simulink library "lib_all"  (Simulink 7.6)
settings: Solver=ode45  SolverMode=SingleTasking  StartTime=0.0  StopTime=10.0  RelTol=1e-3
values from neighbouring files (symbols this model uses):
  map_lib_03 = [6.8679 7.0159 7.4571 8.1849 9.1892 10.456 11.97 13.71 15.655 17.781 20.063 22.474 ... (803 elements, 73x11)]    [scr_simdata.mat]    (also in scr_simdata_all.mat)
  map_lib_06 = [2.5784 2.6727 2.9588 3.4453 4.1444 5.068 6.226 7.6242 9.2634 11.139 13.24 15.552 ... (803 elements, 73x11)]    [scr_simdata.mat]    (also in scr_simdata_all.mat)
  map_lib_09 = [0.0025784 0.0026727 0.0029588 0.0034453 0.0041444 0.005068 0.006226 0.0076242 0.0092634 0.011139 0.01324 0.015552 ... (803 elements, 73x11)]    [scr_simdata.mat]    (also in scr_simdata_all.mat)
  map_lib_10 = [2.74 2.75 2.76 2.77 2.78 2.79 2.8 2.81 2.82 2.83 2.84 2.85 ... (81 elements, 1x81)]    [scr_simdata.mat]    (also in scr_simdata_all.mat)
  map_lib_11 = [1.1983 1.2027 1.207 1.2113 1.2156 1.2198 1.2241 1.2283 1.2326 1.2368 1.241 1.2451 ... (81 elements, 1x81)]    [scr_simdata.mat]    (also in scr_simdata_all.mat)
  map_lib_12 = [2.74 2.75 2.76 2.77 2.78 2.79 2.8 2.81 2.82 2.83 2.84 2.85 ... (81 elements, 1x81)]    [scr_simdata.mat]    (also in scr_simdata_all.mat)
  map_lib_13 = [1.74 1.75 1.76 1.77 1.78 1.79 1.8 1.81 1.82 1.83 1.84 1.85 ... (81 elements, 1x81)]    [scr_simdata.mat]    (also in scr_simdata_all.mat)
  map_lib_16 = [0.00048828125 1.6159 1.8962 2.0821 2.2251 2.3426 2.4433 2.5318 2.611 2.683 2.749 2.8101 ... (1419 elements, 129x11)]    [scr_simdata.mat]    (also in scr_simdata_all.mat)
  map_mr2_01 = [0 800 1600 2400 3200 4000]    [scr_simdata.mat]    (also in scr_simdata_all.mat)
  map_mr2_02 = [1.32 1.32 1.33 1.34 1.36 1.38]    [scr_simdata.mat]    (also in scr_simdata_all.mat)
  map_mr2_03 = [0 800 1600 2400 3200 4000]    [scr_simdata.mat]    (also in scr_simdata_all.mat)
  map_mr2_04 = [1.32 1.32 1.33 1.34 1.36 1.38]    [scr_simdata.mat]    (also in scr_simdata_all.mat)
  map_mr2_05 = [0 800 1600 2400 3200 4000]    [scr_simdata.mat]    (also in scr_simdata_all.mat)
  map_mr2_06 = [1.32 1.32 1.33 1.34 1.36 1.38]    [scr_simdata.mat]    (also in scr_simdata_all.mat)
  Parameter1 = 6    [scr_simdata.mat]    (also in scr_simdata_all.mat)
  Parameter101 = 30    [scr_simdata.mat]    (also in scr_simdata_all.mat)
  Parameter102 = 12    [scr_simdata.mat]    (also in scr_simdata_all.mat)
  Parameter103 = -240    [scr_simdata.mat]    (also in scr_simdata_all.mat)
  Parameter108 = 48    [scr_simdata.mat]    (also in scr_simdata_all.mat)
  Parameter120 = 16    [scr_simdata.mat]    (also in scr_simdata_all.mat)
  Parameter121 = 87    [scr_simdata.mat]    (also in scr_simdata_all.mat)
  Parameter123 = 78    [scr_simdata.mat]    (also in scr_simdata_all.mat)
  Parameter124 = 60    [scr_simdata.mat]    (also in scr_simdata_all.mat)
  Parameter125 = -480    [scr_simdata.mat]    (also in scr_simdata_all.mat)
  Parameter130 = 96    [scr_simdata.mat]    (also in scr_simdata_all.mat)
  Parameter131 = 3    [scr_simdata.mat]    (also in scr_simdata_all.mat)
  Parameter144 = 3    [scr_simdata.mat]    (also in scr_simdata_all.mat)
  Parameter18 = 128    [scr_simdata.mat]    (also in scr_simdata_all.mat)
  Parameter19 = 16    [scr_simdata.mat]    (also in scr_simdata_all.mat)
  Parameter2 = 12    [scr_simdata.mat]    (also in scr_simdata_all.mat)
  Parameter20 = 7    [scr_simdata.mat]    (also in scr_simdata_all.mat)
  Parameter21 = 4    [scr_simdata.mat]    (also in scr_simdata_all.mat)
  Parameter3 = 18    [scr_simdata.mat]    (also in scr_simdata_all.mat)
  Parameter32 = 1    [scr_simdata.mat]    (also in scr_simdata_all.mat)
  Parameter34 = 24    [scr_simdata.mat]    (also in scr_simdata_all.mat)
  Parameter4 = 138    [scr_simdata.mat]    (also in scr_simdata_all.mat)
  Parameter42 = 48    [scr_simdata.mat]    (also in scr_simdata_all.mat)
  Parameter5 = 135    [scr_simdata.mat]    (also in scr_simdata_all.mat)
  Parameter55 = 0    [scr_simdata.mat]    (also in scr_simdata_all.mat)
  Parameter56 = 0    [scr_simdata.mat]    (also in scr_simdata_all.mat)
  Parameter57 = 1    [scr_simdata.mat]    (also in scr_simdata_all.mat)
  Parameter58 = 1    [scr_simdata.mat]    (also in scr_simdata_all.mat)
  Parameter59 = 2    [scr_simdata.mat]    (also in scr_simdata_all.mat)
  Parameter6 = 132    [scr_simdata.mat]    (also in scr_simdata_all.mat)
  Parameter60 = 2    [scr_simdata.mat]    (also in scr_simdata_all.mat)
  Parameter63 = 144    [scr_simdata.mat]    (also in scr_simdata_all.mat)
  Parameter7 = 126    [scr_simdata.mat]    (also in scr_simdata_all.mat)
  Parameter76 = 64    [scr_simdata.mat]    (also in scr_simdata_all.mat)
  Parameter77 = 135    [scr_simdata.mat]    (also in scr_simdata_all.mat)
  Parameter79 = 126    [scr_simdata.mat]    (also in scr_simdata_all.mat)
  Parameter80 = 108    [scr_simdata.mat]    (also in scr_simdata_all.mat)
  Parameter81 = 0    [scr_simdata.mat]    (also in scr_simdata_all.mat)
  Parameter86 = 0    [scr_simdata.mat]    (also in scr_simdata_all.mat)
  Parameter98 = 112    [scr_simdata.mat]    (also in scr_simdata_all.mat)
  Parameter99 = 39    [scr_simdata.mat]    (also in scr_simdata_all.mat)
undefined symbols (not defined in the file or in its folder): cylvolkp_hih_map2h, cylvolkp_map2h, pk1k_map2h
libraries: simulink x2
(part 15 of 36)

== system lib_all/SubSystem7/SubSystem134/SubSystem136/SubSystem139 ==
  Block323 : Inport
  Block324 : Inport  Port=2
  Block325 : Inport  Port=3
  Block326 : Constant  Value=Parameter20  OutDataTypeStr=double  SampleTime=-1
  Block327 : Constant  Value=2  OutDataTypeStr=double  SampleTime=-1
  Block328 : Constant  Value=0  OutDataTypeStr=double  SampleTime=-1
  Block329 : Math  Operator=pow  SaturateOnIntegerOverflow=on
  Block330 : Product  Inputs=*/  OutDataTypeStr=double  SaturateOnIntegerOverflow=on  RndMeth=Zero  InputSameDT=on
  Block331 : Product  Inputs=*/  OutDataTypeStr=double  SaturateOnIntegerOverflow=on  RndMeth=Zero  InputSameDT=on
  Block332 : Sum  Inputs=-+  OutDataTypeStr=double  RndMeth=Zero  SaturateOnIntegerOverflow=on  InputSameDT=on
  SubSystem140 : Subsystem
  SubSystem141 : Subsystem  Priority=1  TreatAsAtomicUnit=on  mask(***ATC.DSWVEC***){bd_version=1.01.A, r0=DSM371, r1=-1, r2=, r3=, r4=, r5=, r6=, r7=, r8=, r9=, c0=, c1=, c2=, c3=, c4=, c5=, c6=, c7=, c8=, c9=}
      mask prompts: bd_version "Version"
      mask help: これはAutoCからのブロックです．編集禁止．
      settings: AttributesFormatString=Attribute62  OpenFcn=warndlg('変更禁止!');
      mask Help: web(ui_blockhelpmng(gcb));
      mask Display: disp('ATC.DSRVEC')
  SubSystem142 : Subsystem  Priority=2  TreatAsAtomicUnit=on  mask(***ATC.DSWVEC***){bd_version=1.01.A, r0=DSM373, r1=-1, r2=, r3=, r4=, r5=, r6=, r7=, r8=, r9=, c0=, c1=, c2=, c3=, c4=, c5=, c6=, c7=, c8=, c9=}
      mask prompts: bd_version "Version"
      mask help: これはAutoCからのブロックです．編集禁止．
      settings: AttributesFormatString=Attribute63  OpenFcn=warndlg('変更禁止!');
      mask Help: web(ui_blockhelpmng(gcb));
      mask Display: disp('ATC.DSRVEC')
  SubSystem143 : Subsystem  Priority=3  TreatAsAtomicUnit=on  mask(***ATC.DSWVEC***){bd_version=1.01.A, r0=DSM375, r1=-1, r2=, r3=, r4=, r5=, r6=, r7=, r8=, r9=, c0=, c1=, c2=, c3=, c4=, c5=, c6=, c7=, c8=, c9=}
      mask prompts: bd_version "Version"
      mask help: これはAutoCからのブロックです．編集禁止．
      settings: AttributesFormatString=Attribute64  OpenFcn=warndlg('変更禁止!');
      mask Help: web(ui_blockhelpmng(gcb));
      mask Display: disp('ATC.DSRVEC')
  SubSystem144 : Subsystem  Priority=4
      settings: AttributesFormatString=Attribute65
  wires:
  Block327/1 -> Block329/1
  Block326/1 -> Block329/2
  Block330/1 -> SubSystem141/1(input), Block332/2
  Block331/1 -> SubSystem142/1(input), Block332/1
  Block323/1 -> Block330/1
  Block325/1 -> SubSystem141/2(element), SubSystem143/2(element), SubSystem142/2(element)
  Block324/1 -> Block331/1
  Block332/1 -> SubSystem143/1(input), SubSystem140/1(In1)
  Block328/1 -> SubSystem140/2(In2)
  SubSystem140/1(Out1) -> SubSystem144/Enable
  Block329/1 -> Block330/2, Block331/2

== system lib_all/SubSystem7/SubSystem134/SubSystem136/SubSystem149 ==
  Block343 : Inport
  Block344 : Inport  Port=2
  Block345 : TriggerPort  TriggerType=function-call
  Block346 : Constant  OutDataTypeStr=double  SampleTime=-1
  Block347 : Sum  OutDataTypeStr=double  RndMeth=Zero  SaturateOnIntegerOverflow=on  InputSameDT=on
  Block348 : Sum  OutDataTypeStr=double  RndMeth=Zero  SaturateOnIntegerOverflow=on  InputSameDT=on
  Block349 : Switch  Threshold=0.5  RndMeth=Zero  SaturateOnIntegerOverflow=on  InputSameDT=on
  Block350 : Switch  Threshold=0.5  RndMeth=Zero  SaturateOnIntegerOverflow=on  InputSameDT=on
  Block351 : UnitDelay  SampleTime=-1
  Block352 : UnitDelay  SampleTime=-1
  SubSystem150 : Subsystem
  SubSystem151 : Subsystem
  SubSystem155 : Subsystem
  Block361 : Outport
  Block362 : Outport  Port=2
  wires:
  Block343/1 -> SubSystem151/2(Block354), SubSystem155/2(Block358), SubSystem150/1(In1)
  Block346/1 -> SubSystem150/2(In2)
  SubSystem150/1(Out1) -> Block349/2, Block350/2
  Block349/1 -> Block361/1, Block351/1
  Block344/1 -> SubSystem151/1(Block353), SubSystem155/1(Block357)
  Block347/1 -> Block349/3
  SubSystem151/1(Block356) -> Block347/1, Block349/1
  Block351/1 -> Block347/2
  Block350/1 -> Block362/1, Block352/1
  Block348/1 -> Block350/3
  SubSystem155/1(Block360) -> Block348/1, Block350/1
  Block352/1 -> Block348/2

== system lib_all/SubSystem7/SubSystem161/SubSystem164/SubSystem165 ==
  note: ***編集禁止***
  N : Inport
  For_loop : Stateflow chart  TreatAsAtomicUnit=on
  action : Outport
  i : Outport  Port=2
  wires:
  For_loop/2(function_call) -> action/1
  N/1 -> For_loop/1(N_Loop)
  For_loop/1(idx) -> i/1

== system lib_all/SubSystem7/SubSystem161/SubSystem164/SubSystem167 ==
  note: ***編集禁止***
  N : Inport
  For_loop : Stateflow chart  TreatAsAtomicUnit=on
  action : Outport
  i : Outport  Port=2
  wires:
  For_loop/2(function_call) -> action/1
  N/1 -> For_loop/1(N_Loop)
  For_loop/1(idx) -> i/1

== system lib_all/SubSystem7/SubSystem161/SubSystem164/SubSystem169 ==
  Block375 : Inport
  Block376 : TriggerPort  TriggerType=function-call
  Block377 : Constant  OutDataTypeStr=double  SampleTime=-1
  Block378 : Product  Inputs=*/  OutDataTypeStr=double  SaturateOnIntegerOverflow=on  RndMeth=Zero  InputSameDT=on
  SubSystem170 : Subsystem  TreatAsAtomicUnit=on  mask(***ATC.DSWVEC***){bd_version=1.01.A, r0=DSM87, r1=-1, r2=, r3=, r4=, r5=, r6=, r7=, r8=, r9=, c0=, c1=, c2=, c3=, c4=, c5=, c6=, c7=, c8=, c9=}
      mask prompts: bd_version "Version"
      mask help: これはAutoCからのブロックです．編集禁止．
      settings: OpenFcn=warndlg('変更禁止!');
      mask Help: web(ui_blockhelpmng(gcb));
      mask Display: disp('ATC.DSRVEC')
  SubSystem171 : Subsystem  TreatAsAtomicUnit=on  mask(***ATC.DSRVEC***){bd_version=1.01.A, r0=DSM375, r1=-1, r2=, r3=, r4=, r5=, r6=, r7=, r8=, r9=, c0=, c1=, c2=, c3=, c4=, c5=, c6=, c7=, c8=, c9=}
      mask prompts: bd_version "Version"
      mask help: これはAutoCからのブロックです．編集禁止．
      settings: OpenFcn=warndlg('変更禁止!');
      mask Help: web(ui_blockhelpmng(gcb));
      mask Display: disp('ATC.DSRVEC')
  SubSystem172 : Subsystem  TreatAsAtomicUnit=on  mask(***ATC.DSRVEC***){bd_version=1.01.A, r0=DSM375, r1=-1, r2=, r3=, r4=, r5=, r6=, r7=, r8=, r9=, c0=, c1=, c2=, c3=, c4=, c5=, c6=, c7=, c8=, c9=}
      mask prompts: bd_version "Version"
      mask help: これはAutoCからのブロックです．編集禁止．
      settings: OpenFcn=warndlg('変更禁止!');
      mask Help: web(ui_blockhelpmng(gcb));
      mask Display: disp('ATC.DSRVEC')
  wires:
  Block377/1 -> SubSystem172/1(element)
  Block375/1 -> SubSystem170/2(element), SubSystem171/1(element)
  SubSystem172/1(Output) -> Block378/1
  SubSystem171/1(Output) -> Block378/2
  Block378/1 -> SubSystem170/1(input)

== system lib_all/SubSystem7/SubSystem161/SubSystem164/SubSystem173 ==
  Block379 : Inport
  Block380 : TriggerPort  TriggerType=function-call
  Block381 : Constant  Value=0  OutDataTypeStr=double  SampleTime=-1
  SubSystem174 : Subsystem  TreatAsAtomicUnit=on  mask(***ATC.DSWVEC***){bd_version=1.01.A, r0=DSM374, r1=-1, r2=, r3=, r4=, r5=, r6=, r7=, r8=, r9=, c0=, c1=, c2=, c3=, c4=, c5=, c6=, c7=, c8=, c9=}
      mask prompts: bd_version "Version"
      mask help: これはAutoCからのブロックです．編集禁止．
      settings: OpenFcn=warndlg('変更禁止!');
      mask Help: web(ui_blockhelpmng(gcb));
      mask Display: disp('ATC.DSRVEC')
  wires:
  Block379/1 -> SubSystem174/2(element)
  Block381/1 -> SubSystem174/1(input)

== system lib_all/SubSystem7/SubSystem161/SubSystem164/SubSystem175 ==
  Block382 : Constant  Value=0  OutDataTypeStr=double  SampleTime=-1
  Block383 : DataStoreWrite  DataStoreName=DSM365
  wires:
  Block382/1 -> Block383/1

== system lib_all/SubSystem7/SubSystem9/SubSystem13/SubSystem120 ==
  note: Text16
  Block283 : Inport
  Block284 : Inport  Port=2
  Block285 : Inport  Port=3
  Block286 : SwitchCase  CaseConditions={1 2 3}
  Block287 : Terminator
  SubSystem121 : Subsystem
      settings: RTWSystemCode=Inline
  SubSystem123 : Subsystem
      settings: RTWSystemCode=Inline
  SubSystem125 : Subsystem
      settings: RTWSystemCode=Inline
  wires:
  Block284/1 -> Block286/1
  Block285/1 -> SubSystem121/2(Block289), SubSystem123/2(Block292), SubSystem125/2(Block295)
  Block283/1 -> SubSystem121/1(Block288), SubSystem123/1(Block291), SubSystem125/1(Block294)
  Block286/1 -> SubSystem121/Action
  Block286/2 -> SubSystem123/Action
  Block286/3 -> SubSystem125/Action
  Block286/4 -> Block287/1

== system lib_all/SubSystem7/SubSystem9/SubSystem13/SubSystem127 ==
  note: Text17
  Block297 : Inport
  Block298 : Inport  Port=2
  Block299 : Inport  Port=3
  Block300 : SwitchCase  CaseConditions={1 2 3}
  Block301 : Terminator
  SubSystem128 : Subsystem
      settings: RTWSystemCode=Inline
  SubSystem130 : Subsystem
      settings: RTWSystemCode=Inline
  SubSystem132 : Subsystem
      settings: RTWSystemCode=Inline
  wires:
  Block300/2 -> SubSystem130/Action
  Block300/1 -> SubSystem128/Action
  Block299/1 -> SubSystem130/2(Block306), SubSystem132/2(Block309), SubSystem128/2(Block303)
  Block297/1 -> SubSystem130/1(Block305), SubSystem132/1(Block308), SubSystem128/1(Block302)
  Block298/1 -> Block300/1
  Block300/3 -> SubSystem132/Action
  Block300/4 -> Block301/1

== system lib_all/SubSystem7/SubSystem9/SubSystem13/SubSystem14 ==
  note: Text9
  Block39 : Inport
  Block40 : Inport  Port=2
  Block41 : Inport  Port=3
  Block42 : Inport  Port=4
  Block43 : Constant  Value=0  OutDataTypeStr=double  SampleTime=-1
  Block44 : Constant  OutDataTypeStr=double  SampleTime=-1
  Block45 : Constant  Value=0  OutDataTypeStr=double  SampleTime=-1
  Block46 : Gain  Gain=1/5  SaturateOnIntegerOverflow=on  OutDataTypeStr=Inherit: Same as input  ParamDataTypeStr=Inherit: Same as input
      settings: AttributesFormatString=Attribute20
  Block47 : Product  OutDataTypeStr=double  SaturateOnIntegerOverflow=on  RndMeth=Zero  InputSameDT=on
  Block48 : Sum  Inputs=-+  OutDataTypeStr=double  RndMeth=Zero  SaturateOnIntegerOverflow=on  InputSameDT=on
  Block49 : Sum  OutDataTypeStr=double  RndMeth=Zero  SaturateOnIntegerOverflow=on  InputSameDT=on
  Block50 : Sum  OutDataTypeStr=double  RndMeth=Zero  SaturateOnIntegerOverflow=on  InputSameDT=on
  Block51 : Switch  Threshold=0.5  RndMeth=Zero  SaturateOnIntegerOverflow=on  InputSameDT=on
  SubSystem16 : Subsystem
  SubSystem17 : Subsystem
  SubSystem23 : Subsystem
  Block160 : Outport
  wires:
  Block50/1 -> SubSystem23/3(Block81), SubSystem17/2(Block53)
  Block39/1 -> Block50/1
  Block46/1 -> Block49/2
  Block47/1 -> Block46/1
  Block44/1 -> Block50/2
  Block43/1 -> Block51/1
  Block51/1 -> Block160/1
  SubSystem16/1(Out1) -> Block51/2
  Block45/1 -> SubSystem16/2(In2)
  Block41/1 -> SubSystem17/3(Block54)
  Block49/1 -> SubSystem16/1(In1), Block51/3
  SubSystem17/2(Block78) -> Block47/2
  Block48/1 -> Block47/1  "SigLabel3"
  SubSystem23/2(Block159) -> Block48/2
  SubSystem23/1(Block158) -> Block48/1, Block49/1
  Block42/1 -> SubSystem23/2(Block80)
  SubSystem17/1(Block77) -> SubSystem23/1(Block79)
  Block40/1 -> SubSystem17/1(Block52)

== system lib_all/SubSystem7/SubSystem9/SubSystem13/SubSystem67 ==
  note: Text15
  Block161 : Inport
  Block162 : Inport  Port=2
  Block163 : Inport  Port=3
  Block164 : Inport  Port=4
  Block165 : Constant  Value=0  OutDataTypeStr=double  SampleTime=-1
  Block166 : Constant  OutDataTypeStr=double  SampleTime=-1
  Block167 : Constant  Value=0  OutDataTypeStr=double  SampleTime=-1
  Block168 : Gain  Gain=1/5  SaturateOnIntegerOverflow=on  OutDataTypeStr=Inherit: Same as input  ParamDataTypeStr=Inherit: Same as input
      settings: AttributesFormatString=Attribute39
  Block169 : Product  OutDataTypeStr=double  SaturateOnIntegerOverflow=on  RndMeth=Zero  InputSameDT=on
  Block170 : Sum  Inputs=-+  OutDataTypeStr=double  RndMeth=Zero  SaturateOnIntegerOverflow=on  InputSameDT=on
  Block171 : Sum  OutDataTypeStr=double  RndMeth=Zero  SaturateOnIntegerOverflow=on  InputSameDT=on
  Block172 : Sum  OutDataTypeStr=double  RndMeth=Zero  SaturateOnIntegerOverflow=on  InputSameDT=on
  Block173 : Switch  Threshold=0.5  RndMeth=Zero  SaturateOnIntegerOverflow=on  InputSameDT=on
  SubSystem69 : Subsystem
  SubSystem70 : Subsystem
  SubSystem76 : Subsystem
  Block282 : Outport
  wires:
  Block172/1 -> SubSystem76/3(Block203), SubSystem70/2(Block175)
  Block161/1 -> Block172/1
  Block168/1 -> Block171/2
  Block169/1 -> Block168/1
  Block166/1 -> Block172/2
  Block165/1 -> Block173/1
  Block173/1 -> Block282/1
  SubSystem69/1(Out1) -> Block173/2
  Block167/1 -> SubSystem69/2(In2)
  Block163/1 -> SubSystem70/3(Block176)
  Block171/1 -> SubSystem69/1(In1), Block173/3
  SubSystem70/2(Block200) -> Block169/2
  Block170/1 -> Block169/1  "SigLabel6"
  SubSystem76/2(Block281) -> Block170/2
  SubSystem76/1(Block280) -> Block170/1, Block171/1
  Block164/1 -> SubSystem76/2(Block202)
  SubSystem70/1(Block199) -> SubSystem76/1(Block201)
  Block162/1 -> SubSystem70/1(Block174)

== system lib_all/SubSystem727/SubSystem740/SubSystem743/SubSystem744 ==
  Block1737 : Inport
  Block1738 : Inport  Port=2
  Block1739 : Constant  Value=Parameter32  OutDataTypeStr=double  SampleTime=-1
  Block1740 : Constant  Value=2  OutDataTypeStr=double  SampleTime=-1
  Block1741 : Constant  Value=Parameter42  OutDataTypeStr=double  SampleTime=-1
  Block1742 : Constant  Value=0  OutDataTypeStr=double  SampleTime=-1
  Block1743 : Constant  Value=72  OutDataTypeStr=double  SampleTime=-1
  Block1744 : Constant  Value=Parameter34  OutDataTypeStr=double  SampleTime=-1
  Block1745 : Logic  Operator=OR
  Block1746 : Sum  Inputs=+-  OutDataTypeStr=double  RndMeth=Zero  SaturateOnIntegerOverflow=on  InputSameDT=on
  Block1747 : Switch  Threshold=0.5  RndMeth=Zero  SaturateOnIntegerOverflow=on  InputSameDT=on
  Block1748 : Switch  Threshold=0.5  RndMeth=Zero  SaturateOnIntegerOverflow=on  InputSameDT=on
  SubSystem745 : Subsystem
  SubSystem746 : Subsystem
  SubSystem747 : Subsystem
      settings: RTWSystemCode=Inline
  Block1755 : Outport
  wires:
  Block1748/1 -> Block1755/1
  Block1747/1 -> Block1746/2
  Block1744/1 -> Block1747/3
  Block1741/1 -> Block1747/1
  Block1745/1 -> Block1748/2
  SubSystem746/1(Out1) -> Block1745/2
  SubSystem745/1(Out1) -> Block1747/2, Block1745/1
  Block1740/1 -> SubSystem746/2(In2)
  Block1739/1 -> SubSystem745/1(In1)
  Block1738/1 -> SubSystem746/1(In1), SubSystem745/2(In2)
  Block1737/1 -> Block1746/1, Block1748/3
  Block1746/1 -> SubSystem747/2(Block1750)
  Block1742/1 -> SubSystem747/3(Block1751)
  Block1743/1 -> SubSystem747/1(Block1749)
  SubSystem747/1(Block1754) -> Block1748/1

== system lib_all/SubSystem727/SubSystem740/SubSystem743/SubSystem749 ==
  Block1756 : Inport
  Block1757 : Inport  Port=2
  Block1758 : Inport  Port=3
  Block1759 : Sum  OutDataTypeStr=double  RndMeth=Zero  SaturateOnIntegerOverflow=on  InputSameDT=on
  Block1760 : Switch  Threshold=0.5  RndMeth=Zero  SaturateOnIntegerOverflow=on  InputSameDT=on
  SubSystem750 : Subsystem
  Block1761 : Outport
  wires:
  Block1756/1 -> Block1759/1
  Block1760/1 -> Block1761/1
  Block1759/1 -> Block1760/1
  SubSystem750/1(Out1) -> Block1760/2
  Block1758/1 -> SubSystem750/2(In2)
  Block1757/1 -> Block1759/2, Block1760/3, SubSystem750/1(In1)

== system lib_all/SubSystem727/SubSystem740/SubSystem752/SubSystem753 ==
  note: ***編集禁止***
  In1 : Inport
  In2 : Inport  Port=2
  RO1 : RelationalOperator  Operator=>  InputSameDT=on
  single1 : DataTypeConversion  OutDataTypeStr=single  SaturateOnIntegerOverflow=on  RndMeth=Zero
  single2 : DataTypeConversion  OutDataTypeStr=single  SaturateOnIntegerOverflow=on  RndMeth=Zero
  Out1 : Outport
  wires:
  RO1/1 -> Out1/1
  In2/1 -> single2/1
  In1/1 -> single1/1
  single1/1 -> RO1/1
  single2/1 -> RO1/2

== system lib_all/SubSystem727/SubSystem754/SubSystem756/SubSystem757 ==
  note: ***編集禁止***
  mult : Inport
  div : Inport  Port=2
  init : Inport  Port=3
  Product : Subsystem  TreatAsAtomicUnit=on
      settings: RTWSystemCode=Inline
  Out : Outport
  wires:
  Product/1(Out) -> Out/1
  mult/1 -> Product/1(mult)
  div/1 -> Product/2(div)
  init/1 -> Product/3(init)

== system lib_all/SubSystem727/SubSystem754/SubSystem756/SubSystem758 ==
  note: ***編集禁止***
  element : Inport
  Inside_DSR : DataStoreRead  DataStoreName=DSM95
  Selector : Selector  IndexOptions=Index vector (port)  Indices=[1 3]  OutputSizes=1
  Output : Outport
  wires:
  Selector/1 -> Output/1
  element/1 -> Selector/2
  Inside_DSR/1 -> Selector/1

== system lib_all/SubSystem727/SubSystem754/SubSystem756/SubSystem759 ==
  Block1804 : Inport
  Block1805 : Inport  Port=2
  Block1806 : Inport  Port=3
  Block1807 : Sum  OutDataTypeStr=double  RndMeth=Zero  SaturateOnIntegerOverflow=on  InputSameDT=on
  Block1808 : Sum  Inputs=+-  OutDataTypeStr=double  RndMeth=Zero  SaturateOnIntegerOverflow=on  InputSameDT=on
  Block1809 : Switch  Threshold=0.5  RndMeth=Zero  SaturateOnIntegerOverflow=on  InputSameDT=on
  Block1810 : Switch  Threshold=0.5  RndMeth=Zero  SaturateOnIntegerOverflow=on  InputSameDT=on
  SubSystem760 : Subsystem
  SubSystem761 : Subsystem
  Block1811 : Outport
  wires:
  Block1805/1 -> SubSystem760/1(In1), Block1808/1, SubSystem761/1(In1), Block1810/3, Block1807/2
  Block1804/1 -> Block1807/1, Block1808/2, SubSystem760/2(In2)
  SubSystem760/1(Out1) -> Block1809/2
  Block1806/1 -> SubSystem761/2(In2)
  SubSystem761/1(Out1) -> Block1810/2
  Block1810/1 -> Block1809/3
  Block1807/1 -> Block1810/1
  Block1809/1 -> Block1811/1
  Block1808/1 -> Block1809/1

== system lib_all/SubSystem727/SubSystem754/SubSystem762/SubSystem763 ==
  note: ***編集禁止***
  In1 : Inport
  In2 : Inport  Port=2
  LO1 : Logic
  RO1 : RelationalOperator  Operator=<  InputSameDT=on
  RO2 : RelationalOperator  Operator=~=  InputSameDT=on
  single1 : DataTypeConversion  OutDataTypeStr=single  SaturateOnIntegerOverflow=on  RndMeth=Zero
  single2 : DataTypeConversion  OutDataTypeStr=single  SaturateOnIntegerOverflow=on  RndMeth=Zero
  Out1 : Outport
  wires:
  LO1/1 -> Out1/1
  RO2/1 -> LO1/2
  RO1/1 -> LO1/1
  In2/1 -> single2/1
  In1/1 -> single1/1
  single1/1 -> RO2/1, RO1/1
  single2/1 -> RO2/2, RO1/2

== system lib_all/SubSystem727/SubSystem754/SubSystem762/SubSystem764 ==
  note: ***編集禁止***
  In1 : Inport
  In2 : Inport  Port=2
  RO1 : RelationalOperator  Operator===  InputSameDT=on
  single1 : DataTypeConversion  OutDataTypeStr=single  SaturateOnIntegerOverflow=on  RndMeth=Zero
  single2 : DataTypeConversion  OutDataTypeStr=single  SaturateOnIntegerOverflow=on  RndMeth=Zero
  Out1 : Outport
  wires:
  RO1/1 -> Out1/1
  In2/1 -> single2/1
  In1/1 -> single1/1
  single1/1 -> RO1/1
  single2/1 -> RO1/2

== system lib_all/SubSystem727/SubSystem754/SubSystem762/SubSystem765 ==
  note: ***編集禁止***
  In1 : Inport
  In2 : Inport  Port=2
  RO1 : RelationalOperator  Operator===  InputSameDT=on
  single1 : DataTypeConversion  OutDataTypeStr=single  SaturateOnIntegerOverflow=on  RndMeth=Zero
  single2 : DataTypeConversion  OutDataTypeStr=single  SaturateOnIntegerOverflow=on  RndMeth=Zero
  Out1 : Outport
  wires:
  RO1/1 -> Out1/1
  In2/1 -> single2/1
  In1/1 -> single1/1
  single1/1 -> RO1/1
  single2/1 -> RO1/2

== system lib_all/SubSystem727/SubSystem754/SubSystem762/SubSystem766 ==
  Block1832 : Inport
  Block1833 : Inport  Port=2
  Block1834 : EnablePort
  Block1835 : Demux  Outputs=2
  Block1836 : Mux  Inputs=2
  SubSystem767 : Subsystem
  SubSystem771 : Subsystem
  Block1845 : Outport
  wires:
  Block1832/1 -> Block1835/1
  Block1833/1 -> SubSystem771/1(Block1841), SubSystem767/1(Block1837)
  Block1835/1 -> SubSystem767/2(Block1838)
  Block1835/2 -> SubSystem771/2(Block1842)
  SubSystem767/1(Block1840) -> Block1836/1
  SubSystem771/1(Block1844) -> Block1836/2
  Block1836/1 -> Block1845/1

== system lib_all/SubSystem727/SubSystem754/SubSystem762/SubSystem775 ==
  Block1846 : Inport
  Block1847 : Inport  Port=2
  Block1848 : EnablePort
  Block1849 : Demux  Outputs=2
  Block1850 : Mux  Inputs=2
  Block1851 : Sum  OutDataTypeStr=double  RndMeth=Zero  SaturateOnIntegerOverflow=on  InputSameDT=on
  SubSystem776 : Subsystem  TreatAsAtomicUnit=on  mask(***ATC.DSRVEC***){bd_version=1.01.A, r0=DSM4, r1=-1, r2=, r3=, r4=, r5=, r6=, r7=, r8=, r9=, c0=, c1=, c2=, c3=, c4=, c5=, c6=, c7=, c8=, c9=}
      mask prompts: bd_version "Version"
      mask help: これはAutoCからのブロックです．編集禁止．
      settings: OpenFcn=warndlg('変更禁止!');
      mask Help: web(ui_blockhelpmng(gcb));
      mask Display: disp('ATC.DSRVEC')
  SubSystem777 : Subsystem
  SubSystem781 : Subsystem
  Block1860 : Outport
  wires:
  Block1849/2 -> SubSystem781/2(Block1857)
  Block1849/1 -> SubSystem777/2(Block1853)
  Block1846/1 -> Block1849/1
  SubSystem777/1(Block1855) -> Block1850/1
  SubSystem781/1(Block1859) -> Block1850/2
  Block1851/1 -> Block1860/1
  SubSystem776/1(Output) -> Block1851/1
  Block1850/1 -> Block1851/2
  Block1847/1 -> SubSystem781/1(Block1856), SubSystem777/1(Block1852), SubSystem776/1(element)

== system lib_all/SubSystem727/SubSystem754/SubSystem762/SubSystem785 ==
  Block1861 : Inport
  Block1862 : Inport  Port=2
  Block1863 : EnablePort
  Block1864 : Demux  Outputs=2
  Block1865 : Mux  Inputs=2
  Block1866 : Sum  OutDataTypeStr=double  RndMeth=Zero  SaturateOnIntegerOverflow=on  InputSameDT=on
  SubSystem786 : Subsystem  TreatAsAtomicUnit=on  mask(***ATC.DSRVEC***){bd_version=1.01.A, r0=DSM2, r1=-1, r2=, r3=, r4=, r5=, r6=, r7=, r8=, r9=, c0=, c1=, c2=, c3=, c4=, c5=, c6=, c7=, c8=, c9=}
      mask prompts: bd_version "Version"
      mask help: これはAutoCからのブロックです．編集禁止．
      settings: OpenFcn=warndlg('変更禁止!');
      mask Help: web(ui_blockhelpmng(gcb));
      mask Display: disp('ATC.DSRVEC')
  SubSystem787 : Subsystem
  SubSystem791 : Subsystem
  Block1875 : Outport
  wires:
  Block1861/1 -> Block1864/1
  Block1864/1 -> SubSystem787/2(Block1868)
  Block1864/2 -> SubSystem791/2(Block1872)
  SubSystem787/1(Block1870) -> Block1865/1
  SubSystem791/1(Block1874) -> Block1865/2
  Block1866/1 -> Block1875/1
  Block1865/1 -> Block1866/2
  Block1862/1 -> SubSystem791/1(Block1871), SubSystem787/1(Block1867), SubSystem786/1(element)
  SubSystem786/1(Output) -> Block1866/1

== system lib_all/SubSystem800/SubSystem803/SubSystem804/SubSystem805 ==
  note: ***編集禁止***
  In1 : Inport
  In2 : Inport  Port=2
  RO1 : RelationalOperator  Operator=>  InputSameDT=on
  single1 : DataTypeConversion  OutDataTypeStr=single  SaturateOnIntegerOverflow=on  RndMeth=Zero
  single2 : DataTypeConversion  OutDataTypeStr=single  SaturateOnIntegerOverflow=on  RndMeth=Zero
  Out1 : Outport
  wires:
  single2/1 -> RO1/2
  single1/1 -> RO1/1
  In1/1 -> single1/1
  In2/1 -> single2/1
  RO1/1 -> Out1/1

== system lib_all/SubSystem800/SubSystem803/SubSystem804/SubSystem806 ==
  note: ***編集禁止***
  In1 : Inport
  In2 : Inport  Port=2
  RO1 : RelationalOperator  Operator=>  InputSameDT=on
  single1 : DataTypeConversion  OutDataTypeStr=single  SaturateOnIntegerOverflow=on  RndMeth=Zero
  single2 : DataTypeConversion  OutDataTypeStr=single  SaturateOnIntegerOverflow=on  RndMeth=Zero
  Out1 : Outport
  wires:
  RO1/1 -> Out1/1
  In2/1 -> single2/1
  In1/1 -> single1/1
  single1/1 -> RO1/1
  single2/1 -> RO1/2

== system lib_all/SubSystem800/SubSystem803/SubSystem804/SubSystem807 ==
  note: ***編集禁止***
  In1 : Inport
  In2 : Inport  Port=2
  LO1 : Logic
  RO1 : RelationalOperator  Operator=<  InputSameDT=on
  RO2 : RelationalOperator  Operator=~=  InputSameDT=on
  single1 : DataTypeConversion  OutDataTypeStr=single  SaturateOnIntegerOverflow=on  RndMeth=Zero
  single2 : DataTypeConversion  OutDataTypeStr=single  SaturateOnIntegerOverflow=on  RndMeth=Zero
  Out1 : Outport
  wires:
  LO1/1 -> Out1/1
  RO2/1 -> LO1/2
  RO1/1 -> LO1/1
  In2/1 -> single2/1
  In1/1 -> single1/1
  single1/1 -> RO2/1, RO1/1
  single2/1 -> RO2/2, RO1/2

== system lib_all/SubSystem800/SubSystem803/SubSystem804/SubSystem808 ==
  note: ***編集禁止***
  In1 : Inport
  In2 : Inport  Port=2
  LO1 : Logic
  RO1 : RelationalOperator  Operator=<  InputSameDT=on
  RO2 : RelationalOperator  Operator=~=  InputSameDT=on
  single1 : DataTypeConversion  OutDataTypeStr=single  SaturateOnIntegerOverflow=on  RndMeth=Zero
  single2 : DataTypeConversion  OutDataTypeStr=single  SaturateOnIntegerOverflow=on  RndMeth=Zero
  Out1 : Outport
  wires:
  LO1/1 -> Out1/1
  RO2/1 -> LO1/2
  RO1/1 -> LO1/1
  In2/1 -> single2/1
  In1/1 -> single1/1
  single1/1 -> RO2/1, RO1/1
  single2/1 -> RO2/2, RO1/2

== system lib_all/SubSystem800/SubSystem803/SubSystem809/SubSystem810 ==
  note: ***編集禁止***
  In1 : Inport
  In2 : Inport  Port=2
  RO1 : RelationalOperator  Operator=>  InputSameDT=on
  single1 : DataTypeConversion  OutDataTypeStr=single  SaturateOnIntegerOverflow=on  RndMeth=Zero
  single2 : DataTypeConversion  OutDataTypeStr=single  SaturateOnIntegerOverflow=on  RndMeth=Zero
  Out1 : Outport
  wires:
  single2/1 -> RO1/2
  single1/1 -> RO1/1
  In1/1 -> single1/1
  In2/1 -> single2/1
  RO1/1 -> Out1/1

== system lib_all/SubSystem800/SubSystem803/SubSystem809/SubSystem811 ==
  note: ***編集禁止***
  In1 : Inport
  In2 : Inport  Port=2
  RO1 : RelationalOperator  Operator=>  InputSameDT=on
  single1 : DataTypeConversion  OutDataTypeStr=single  SaturateOnIntegerOverflow=on  RndMeth=Zero
  single2 : DataTypeConversion  OutDataTypeStr=single  SaturateOnIntegerOverflow=on  RndMeth=Zero
  Out1 : Outport
  wires:
  RO1/1 -> Out1/1
  In2/1 -> single2/1
  In1/1 -> single1/1
  single1/1 -> RO1/1
  single2/1 -> RO1/2

== system lib_all/SubSystem800/SubSystem803/SubSystem809/SubSystem812 ==
  note: ***編集禁止***
  In1 : Inport
  In2 : Inport  Port=2
  LO1 : Logic
  RO1 : RelationalOperator  Operator=<  InputSameDT=on
  RO2 : RelationalOperator  Operator=~=  InputSameDT=on
  single1 : DataTypeConversion  OutDataTypeStr=single  SaturateOnIntegerOverflow=on  RndMeth=Zero
  single2 : DataTypeConversion  OutDataTypeStr=single  SaturateOnIntegerOverflow=on  RndMeth=Zero
  Out1 : Outport
  wires:
  LO1/1 -> Out1/1
  RO2/1 -> LO1/2
  RO1/1 -> LO1/1
  In2/1 -> single2/1
  In1/1 -> single1/1
  single1/1 -> RO2/1, RO1/1
  single2/1 -> RO2/2, RO1/2

== system lib_all/SubSystem800/SubSystem803/SubSystem809/SubSystem813 ==
  note: ***編集禁止***
  In1 : Inport
  In2 : Inport  Port=2
  LO1 : Logic
  RO1 : RelationalOperator  Operator=<  InputSameDT=on
  RO2 : RelationalOperator  Operator=~=  InputSameDT=on
  single1 : DataTypeConversion  OutDataTypeStr=single  SaturateOnIntegerOverflow=on  RndMeth=Zero
  single2 : DataTypeConversion  OutDataTypeStr=single  SaturateOnIntegerOverflow=on  RndMeth=Zero
  Out1 : Outport
  wires:
  LO1/1 -> Out1/1
  RO2/1 -> LO1/2
  RO1/1 -> LO1/1
  In2/1 -> single2/1
  In1/1 -> single1/1
  single1/1 -> RO2/1, RO1/1
  single2/1 -> RO2/2, RO1/2

== system lib_all/SubSystem800/SubSystem803/SubSystem814/SubSystem815 ==
  note: ***編集禁止***
  In1 : Inport
  In2 : Inport  Port=2
  RO1 : RelationalOperator  Operator=>  InputSameDT=on
  single1 : DataTypeConversion  OutDataTypeStr=single  SaturateOnIntegerOverflow=on  RndMeth=Zero
  single2 : DataTypeConversion  OutDataTypeStr=single  SaturateOnIntegerOverflow=on  RndMeth=Zero
  Out1 : Outport
  wires:
  single2/1 -> RO1/2
  single1/1 -> RO1/1
  In1/1 -> single1/1
  In2/1 -> single2/1
  RO1/1 -> Out1/1

== system lib_all/SubSystem800/SubSystem803/SubSystem814/SubSystem816 ==
  note: ***編集禁止***
  In1 : Inport
  In2 : Inport  Port=2
  RO1 : RelationalOperator  Operator=>  InputSameDT=on
  single1 : DataTypeConversion  OutDataTypeStr=single  SaturateOnIntegerOverflow=on  RndMeth=Zero
  single2 : DataTypeConversion  OutDataTypeStr=single  SaturateOnIntegerOverflow=on  RndMeth=Zero
  Out1 : Outport
  wires:
  RO1/1 -> Out1/1
  In2/1 -> single2/1
  In1/1 -> single1/1
  single1/1 -> RO1/1
  single2/1 -> RO1/2

== system lib_all/SubSystem800/SubSystem803/SubSystem814/SubSystem817 ==
  note: ***編集禁止***
  In1 : Inport
  In2 : Inport  Port=2
  LO1 : Logic
  RO1 : RelationalOperator  Operator=<  InputSameDT=on
  RO2 : RelationalOperator  Operator=~=  InputSameDT=on
  single1 : DataTypeConversion  OutDataTypeStr=single  SaturateOnIntegerOverflow=on  RndMeth=Zero
  single2 : DataTypeConversion  OutDataTypeStr=single  SaturateOnIntegerOverflow=on  RndMeth=Zero
  Out1 : Outport
  wires:
  LO1/1 -> Out1/1
  RO2/1 -> LO1/2
  RO1/1 -> LO1/1
  In2/1 -> single2/1
  In1/1 -> single1/1
  single1/1 -> RO2/1, RO1/1
  single2/1 -> RO2/2, RO1/2

== system lib_all/SubSystem800/SubSystem803/SubSystem814/SubSystem818 ==
  note: ***編集禁止***
  In1 : Inport
  In2 : Inport  Port=2
  LO1 : Logic
  RO1 : RelationalOperator  Operator=<  InputSameDT=on
  RO2 : RelationalOperator  Operator=~=  InputSameDT=on
  single1 : DataTypeConversion  OutDataTypeStr=single  SaturateOnIntegerOverflow=on  RndMeth=Zero
  single2 : DataTypeConversion  OutDataTypeStr=single  SaturateOnIntegerOverflow=on  RndMeth=Zero
  Out1 : Outport
  wires:
  LO1/1 -> Out1/1
  RO2/1 -> LO1/2
  RO1/1 -> LO1/1
  In2/1 -> single2/1
  In1/1 -> single1/1
  single1/1 -> RO2/1, RO1/1
  single2/1 -> RO2/2, RO1/2

== system lib_all/SubSystem800/SubSystem819/SubSystem832/SubSystem834 ==
  note: ***編集禁止***
  In1 : Inport
  In2 : Inport  Port=2
  RO1 : RelationalOperator  Operator===  InputSameDT=on
  single1 : DataTypeConversion  OutDataTypeStr=single  SaturateOnIntegerOverflow=on  RndMeth=Zero
  single2 : DataTypeConversion  OutDataTypeStr=single  SaturateOnIntegerOverflow=on  RndMeth=Zero
  Out1 : Outport
  wires:
  RO1/1 -> Out1/1
  In2/1 -> single2/1
  In1/1 -> single1/1
  single1/1 -> RO1/1
  single2/1 -> RO1/2

== system lib_all/SubSystem800/SubSystem819/SubSystem832/SubSystem835 ==
  note: Text138
  Block2080 : Inport
  Block2081 : Inport  Port=2
  Block2082 : Constant  Value=0  OutDataTypeStr=double  SampleTime=-1
  Block2083 : Constant  Value=720  OutDataTypeStr=double  SampleTime=-1
  Block2084 : Gain  Gain=5  SaturateOnIntegerOverflow=on  OutDataTypeStr=Inherit: Same as input  ParamDataTypeStr=Inherit: Same as input
      settings: AttributesFormatString=Attribute428
  SubSystem836 : Subsystem
      settings: RTWFcnNameOpts=Use subsystem name
  SubSystem841 : Subsystem
      settings: RTWSystemCode=Inline
  Block2110 : Outport
  wires:
  Block2084/1 -> SubSystem841/2(Block2105)
  SubSystem836/1(Block2103) -> Block2084/1
  Block2081/1 -> SubSystem836/2(Block2086)
  Block2080/1 -> SubSystem836/1(Block2085)
  SubSystem841/1(Block2109) -> Block2110/1
  Block2083/1 -> SubSystem841/1(Block2104)
  Block2082/1 -> SubSystem841/3(Block2106)

== system lib_all/SubSystem800/SubSystem819/SubSystem832/SubSystem843 ==
  Block2111 : Inport
  Block2112 : Inport  Port=2
  Block2113 : Inport  Port=3
  Block2114 : EnablePort
  Block2115 : DataStoreWrite  DataStoreName=DSM12
  Block2116 : DataStoreWrite  DataStoreName=DSM14
  Block2117 : DataStoreWrite  DataStoreName=DSM13
  wires:
  Block2111/1 -> Block2115/1
  Block2113/1 -> Block2117/1
  Block2112/1 -> Block2116/1

== system lib_all/SubSystem800/SubSystem819/SubSystem832/SubSystem844 ==
  note: Text139
  Block2118 : Inport
  Block2119 : Inport  Port=2
  Block2120 : Constant  Value=360  OutDataTypeStr=double  SampleTime=-1
  Block2121 : Constant  Value=360  OutDataTypeStr=double  SampleTime=-1
  Block2122 : Sum  Inputs=+-  OutDataTypeStr=double  RndMeth=Zero  SaturateOnIntegerOverflow=on  InputSameDT=on
  Block2123 : Switch  Threshold=0.5  RndMeth=Zero  SaturateOnIntegerOverflow=on  InputSameDT=on
  Block2124 : Lookup2D  RowIndex=map_lib_05  ColumnIndex=map_lib_04  Table=map_lib_06  SaturateOnIntegerOverflow=on  InputSameDT=on
      settings: AttributesFormatString=<%<OutputValues>>\nAttribute437
  SubSystem845 : Subsystem
  Block2125 : Outport
  wires:
  Block2119/1 -> SubSystem845/1(In1), Block2123/3, Block2122/1
  Block2121/1 -> SubSystem845/2(In2)
  SubSystem845/1(Out1) -> Block2123/2
  Block2122/1 -> Block2123/1
  Block2120/1 -> Block2122/2
  Block2124/1 -> Block2125/1
  Block2118/1 -> Block2124/2
  Block2123/1 -> Block2124/1  "SigLabel56"

== system lib_all/SubSystem800/SubSystem819/SubSystem846/SubSystem848 ==
  note: Text143
  note: Text142
  Block2136 : Inport
  Block2137 : Inport  Port=2
  Block2138 : Constant  Value=0  OutDataTypeStr=double  SampleTime=-1
  Block2139 : Constant  Value=0  OutDataTypeStr=double  SampleTime=-1
  Block2140 : Constant  Value=720  OutDataTypeStr=double  SampleTime=-1
  Block2141 : Constant  Value=5  OutDataTypeStr=double  SampleTime=-1
  Block2142 : DataStoreRead  DataStoreName=DSM50
  Block2143 : DataStoreRead  DataStoreName=DSM35
  Block2144 : Gain  Gain=5  SaturateOnIntegerOverflow=on  OutDataTypeStr=Inherit: Same as input  ParamDataTypeStr=Inherit: Same as input
      settings: AttributesFormatString=Attribute445
  Block2145 : Gain  Gain=5  SaturateOnIntegerOverflow=on  OutDataTypeStr=Inherit: Same as input  ParamDataTypeStr=Inherit: Same as input
      settings: AttributesFormatString=Attribute446
  Block2146 : Gain  Gain=1/5  SaturateOnIntegerOverflow=on  OutDataTypeStr=Inherit: Same as input  ParamDataTypeStr=Inherit: Same as input
      settings: AttributesFormatString=Attribute447
  Block2147 : Product  Inputs=*/  OutDataTypeStr=double  SaturateOnIntegerOverflow=on  RndMeth=Zero  InputSameDT=on
  Block2148 : Rounding
  Block2149 : Rounding
  Block2150 : Sum  Inputs=+-+  OutDataTypeStr=double  RndMeth=Zero  SaturateOnIntegerOverflow=on  InputSameDT=on
  Block2151 : Sum  Inputs=-+  OutDataTypeStr=double  RndMeth=Zero  SaturateOnIntegerOverflow=on  InputSameDT=on
  SubSystem849 : Subsystem  TreatAsAtomicUnit=on  mask(***ATC.DIVISION***){bd_version=1.01.A, r0=チェック有り, r1=, r2=, r3=, r4=, r5=, r6=, r7=, r8=, r9=, c0=, c1=, c2=, c3=, c4=, c5=, c6=, c7=, c8=, c9=}
      mask prompts: bd_version "Version"
      mask help: これはAutoCからのブロックです．編集禁止．
      settings: OpenFcn=warndlg('変更禁止!');
      mask Help: web(ui_blockhelpmng(gcb));
  SubSystem850 : Subsystem  TreatAsAtomicUnit=on  mask(***ATC.DSRVEC***){bd_version=1.01.A, r0=DSM95, r1=-1, r2=, r3=, r4=, r5=, r6=, r7=, r8=, r9=, c0=, c1=, c2=, c3=, c4=, c5=, c6=, c7=, c8=, c9=}
      mask prompts: bd_version "Version"
      mask help: これはAutoCからのブロックです．編集禁止．
      settings: OpenFcn=warndlg('変更禁止!');
      mask Help: web(ui_blockhelpmng(gcb));
      mask Display: disp('ATC.DSRVEC')
  SubSystem851 : Subsystem
      settings: RTWSystemCode=Inline
  Block2160 : Outport
  Block2161 : Outport  Port=2
  wires:
  Block2149/1 -> Block2160/1
  Block2146/1 -> Block2149/1
  Block2145/1 -> Block2150/3
  Block2142/1 -> Block2150/2
  Block2143/1 -> SubSystem849/2(div)
  SubSystem850/1(Output) -> SubSystem849/1(mult)
  Block2138/1 -> SubSystem849/3(init)
  Block2141/1 -> Block2147/2
  Block2151/1 -> Block2161/1
  Block2137/1 -> SubSystem850/1(element)
  Block2140/1 -> SubSystem851/1(Block2152)
  Block2150/1 -> SubSystem851/2(Block2153)  "SigLabel58"
  Block2139/1 -> SubSystem851/3(Block2154)
  SubSystem851/1(Block2159) -> Block2147/1, Block2146/1, Block2151/2
  Block2147/1 -> Block2148/1
  Block2148/1 -> Block2144/1
  Block2144/1 -> Block2151/1
  Block2136/1 -> Block2145/1
  SubSystem849/1(Out) -> Block2150/1  "SigLabel57"

== system lib_all/SubSystem800/SubSystem819/SubSystem846/SubSystem854 ==
  note: Text146
  note: Text145
  note: Text144
  Block2162 : Inport
  Block2163 : Inport  Port=2
  Block2164 : Inport  Port=3
  Block2165 : Constant  Value=0  OutDataTypeStr=double  SampleTime=-1
  Block2166 : Constant  OutDataTypeStr=double  SampleTime=-1
  Block2167 : Constant  OutDataTypeStr=double  SampleTime=-1
  Block2168 : Constant  Value=0  OutDataTypeStr=double  SampleTime=-1
  Block2169 : Constant  OutDataTypeStr=double  SampleTime=-1
  Block2170 : Constant  Value=143  OutDataTypeStr=double  SampleTime=-1
  Block2171 : Demux  Outputs=2
  Block2172 : Logic  Operator=NOT
  Block2173 : Mux  Inputs=2
  Block2174 : Mux  Inputs=2
  Block2175 : Sum  OutDataTypeStr=double  RndMeth=Zero  SaturateOnIntegerOverflow=on  InputSameDT=on
  Block2176 : Sum  OutDataTypeStr=double  RndMeth=Zero  SaturateOnIntegerOverflow=on  InputSameDT=on
  Block2177 : Switch  Threshold=0.5  RndMeth=Zero  SaturateOnIntegerOverflow=on  InputSameDT=on
  Block2178 : Switch  Threshold=0.5  RndMeth=Zero  SaturateOnIntegerOverflow=on  InputSameDT=on
  Block2179 : Switch  Threshold=0.5  RndMeth=Zero  SaturateOnIntegerOverflow=on  InputSameDT=on
  SubSystem855 : Subsystem
  SubSystem856 : Subsystem
  SubSystem857 : Subsystem
  SubSystem858 : Subsystem  TreatAsAtomicUnit=on
  SubSystem867 : Subsystem  TreatAsAtomicUnit=on
  SubSystem877 : Subsystem  TreatAsAtomicUnit=on
  Block2224 : Outport
  Block2225 : Outport  Port=2
  wires:
  Block2162/1 -> SubSystem855/1(In1), Block2175/2, Block2173/1
  Block2170/1 -> SubSystem855/2(In2), Block2174/1
  Block2163/1 -> SubSystem856/1(In1), SubSystem857/1(In1)
  Block2165/1 -> SubSystem856/2(In2)
  SubSystem856/1(Out1) -> Block2177/2, SubSystem858/Enable
  SubSystem858/1(Block2193) -> Block2177/1
  Block2166/1 -> SubSystem857/2(In2)
  SubSystem857/1(Out1) -> Block2178/2, SubSystem867/Enable, Block2172/1
  SubSystem867/1(Block2208) -> Block2178/1
  Block2172/1 -> SubSystem877/Enable
  Block2178/1 -> Block2177/3
  SubSystem877/1(Block2223) -> Block2178/3
  Block2171/1 -> Block2224/1
  Block2171/2 -> Block2225/1
  Block2177/1 -> Block2171/1
  SubSystem855/1(Out1) -> Block2179/2
  Block2167/1 -> Block2175/1
  Block2168/1 -> Block2174/2
  Block2174/1 -> Block2179/3
  Block2175/1 -> Block2173/2
  Block2173/1 -> Block2179/1
  Block2164/1 -> SubSystem858/2(Block2181), SubSystem867/2(Block2195), SubSystem877/2(Block2210)
  Block2179/1 -> Block2176/2
  Block2169/1 -> Block2176/1
  Block2176/1 -> SubSystem858/1(Block2180), SubSystem867/1(Block2194), SubSystem877/1(Block2209)

== system lib_all/SubSystem800/SubSystem887/SubSystem900/SubSystem902 ==
  note: ***編集禁止***
  In1 : Inport
  In2 : Inport  Port=2
  RO1 : RelationalOperator  Operator===  InputSameDT=on
  single1 : DataTypeConversion  OutDataTypeStr=single  SaturateOnIntegerOverflow=on  RndMeth=Zero
  single2 : DataTypeConversion  OutDataTypeStr=single  SaturateOnIntegerOverflow=on  RndMeth=Zero
  Out1 : Outport
  wires:
  RO1/1 -> Out1/1
  In2/1 -> single2/1
  In1/1 -> single1/1
  single1/1 -> RO1/1
  single2/1 -> RO1/2

== system lib_all/SubSystem800/SubSystem887/SubSystem900/SubSystem903 ==
  note: Text157
  Block2305 : Inport
  Block2306 : Inport  Port=2
  Block2307 : Constant  Value=0  OutDataTypeStr=double  SampleTime=-1
  Block2308 : Constant  Value=720  OutDataTypeStr=double  SampleTime=-1
  Block2309 : Gain  Gain=5  SaturateOnIntegerOverflow=on  OutDataTypeStr=Inherit: Same as input  ParamDataTypeStr=Inherit: Same as input
      settings: AttributesFormatString=Attribute465
  SubSystem904 : Subsystem
      settings: RTWFcnNameOpts=Use subsystem name
  SubSystem909 : Subsystem
      settings: RTWSystemCode=Inline
  Block2335 : Outport
  wires:
  Block2309/1 -> SubSystem909/2(Block2330)
  SubSystem904/1(Block2328) -> Block2309/1
  Block2306/1 -> SubSystem904/2(Block2311)
  Block2305/1 -> SubSystem904/1(Block2310)
  SubSystem909/1(Block2334) -> Block2335/1
  Block2308/1 -> SubSystem909/1(Block2329)
  Block2307/1 -> SubSystem909/3(Block2331)

== system lib_all/SubSystem800/SubSystem887/SubSystem900/SubSystem911 ==
  Block2336 : Inport
  Block2337 : Inport  Port=2
  Block2338 : Inport  Port=3
  Block2339 : EnablePort
  Block2340 : DataStoreWrite  DataStoreName=DSM12
  Block2341 : DataStoreWrite  DataStoreName=DSM14
  Block2342 : DataStoreWrite  DataStoreName=DSM13
  wires:
  Block2336/1 -> Block2340/1
  Block2338/1 -> Block2342/1
  Block2337/1 -> Block2341/1

== system lib_all/SubSystem800/SubSystem887/SubSystem900/SubSystem912 ==
  note: Text158
  Block2343 : Inport
  Block2344 : Inport  Port=2
  Block2345 : Constant  Value=360  OutDataTypeStr=double  SampleTime=-1
  Block2346 : Constant  Value=360  OutDataTypeStr=double  SampleTime=-1
  Block2347 : Sum  Inputs=+-  OutDataTypeStr=double  RndMeth=Zero  SaturateOnIntegerOverflow=on  InputSameDT=on
  Block2348 : Switch  Threshold=0.5  RndMeth=Zero  SaturateOnIntegerOverflow=on  InputSameDT=on
  Block2349 : Lookup2D  RowIndex=map_lib_05  ColumnIndex=map_lib_04  Table=map_lib_06  SaturateOnIntegerOverflow=on  InputSameDT=on
      settings: AttributesFormatString=<%<OutputValues>>\nAttribute474
  SubSystem913 : Subsystem
  Block2350 : Outport
  wires:
  Block2344/1 -> SubSystem913/1(In1), Block2348/3, Block2347/1
  Block2346/1 -> SubSystem913/2(In2)
  SubSystem913/1(Out1) -> Block2348/2
  Block2347/1 -> Block2348/1
  Block2345/1 -> Block2347/2
  Block2349/1 -> Block2350/1
  Block2343/1 -> Block2349/2
  Block2348/1 -> Block2349/1  "SigLabel66"

== system lib_all/SubSystem800/SubSystem887/SubSystem914/SubSystem916 ==
  note: Text162
  note: Text161
  Block2361 : Inport
  Block2362 : Inport  Port=2
  Block2363 : Constant  Value=0  OutDataTypeStr=double  SampleTime=-1
  Block2364 : Constant  Value=0  OutDataTypeStr=double  SampleTime=-1
  Block2365 : Constant  Value=720  OutDataTypeStr=double  SampleTime=-1
  Block2366 : Constant  Value=5  OutDataTypeStr=double  SampleTime=-1
  Block2367 : DataStoreRead  DataStoreName=DSM50
  Block2368 : DataStoreRead  DataStoreName=DSM35
  Block2369 : Gain  Gain=5  SaturateOnIntegerOverflow=on  OutDataTypeStr=Inherit: Same as input  ParamDataTypeStr=Inherit: Same as input
      settings: AttributesFormatString=Attribute482
  Block2370 : Gain  Gain=5  SaturateOnIntegerOverflow=on  OutDataTypeStr=Inherit: Same as input  ParamDataTypeStr=Inherit: Same as input
      settings: AttributesFormatString=Attribute483
  Block2371 : Gain  Gain=1/5  SaturateOnIntegerOverflow=on  OutDataTypeStr=Inherit: Same as input  ParamDataTypeStr=Inherit: Same as input
      settings: AttributesFormatString=Attribute484
  Block2372 : Product  Inputs=*/  OutDataTypeStr=double  SaturateOnIntegerOverflow=on  RndMeth=Zero  InputSameDT=on
  Block2373 : Rounding
  Block2374 : Rounding
  Block2375 : Sum  Inputs=+-+  OutDataTypeStr=double  RndMeth=Zero  SaturateOnIntegerOverflow=on  InputSameDT=on
  Block2376 : Sum  Inputs=-+  OutDataTypeStr=double  RndMeth=Zero  SaturateOnIntegerOverflow=on  InputSameDT=on
  SubSystem917 : Subsystem  TreatAsAtomicUnit=on  mask(***ATC.DIVISION***){bd_version=1.01.A, r0=チェック有り, r1=, r2=, r3=, r4=, r5=, r6=, r7=, r8=, r9=, c0=, c1=, c2=, c3=, c4=, c5=, c6=, c7=, c8=, c9=}
      mask prompts: bd_version "Version"
      mask help: これはAutoCからのブロックです．編集禁止．
      settings: OpenFcn=warndlg('変更禁止!');
      mask Help: web(ui_blockhelpmng(gcb));
  SubSystem918 : Subsystem  TreatAsAtomicUnit=on  mask(***ATC.DSRVEC***){bd_version=1.01.A, r0=DSM95, r1=-1, r2=, r3=, r4=, r5=, r6=, r7=, r8=, r9=, c0=, c1=, c2=, c3=, c4=, c5=, c6=, c7=, c8=, c9=}
      mask prompts: bd_version "Version"
      mask help: これはAutoCからのブロックです．編集禁止．
      settings: OpenFcn=warndlg('変更禁止!');
      mask Help: web(ui_blockhelpmng(gcb));
      mask Display: disp('ATC.DSRVEC')
  SubSystem919 : Subsystem
      settings: RTWSystemCode=Inline
  Block2385 : Outport
  Block2386 : Outport  Port=2
  wires:
  Block2374/1 -> Block2385/1
  Block2371/1 -> Block2374/1
  Block2370/1 -> Block2375/3
  Block2367/1 -> Block2375/2
  Block2368/1 -> SubSystem917/2(div)
  SubSystem918/1(Output) -> SubSystem917/1(mult)
  Block2363/1 -> SubSystem917/3(init)
  Block2366/1 -> Block2372/2
  Block2376/1 -> Block2386/1
  Block2362/1 -> SubSystem918/1(element)
  Block2365/1 -> SubSystem919/1(Block2377)
  Block2375/1 -> SubSystem919/2(Block2378)  "SigLabel68"
  Block2364/1 -> SubSystem919/3(Block2379)
  SubSystem919/1(Block2384) -> Block2372/1, Block2371/1, Block2376/2
  Block2372/1 -> Block2373/1
  Block2373/1 -> Block2369/1
  Block2369/1 -> Block2376/1
  Block2361/1 -> Block2370/1
  SubSystem917/1(Out) -> Block2375/1  "SigLabel67"
